simulink model "TosAeb"  (R2021a)
settings: Solver=FixedStepDiscrete  StartTime=0.0  StopTime=inf  FixedStep=0.01  RelTol=1e-3
undefined symbols (not defined in the file or in its folder): ACCModSts, AccSts1, AEB_ObjectMovingOrientation, AEB_ObjectMovingState, CN_BTCActive, CN_CurrentGearR, CN_CurrentGearR_DHT, CN_PTCActive, CN_VDCActive, CN_VSC_EspOff, CN_VSC_FCW_AEB_Error, CN_VSC_FCW_AEB_NoDispay, CN_VSC_FCW_AEB_PerfmDgdtn, CN_VSC_FCW_AEB_TempUnAvlbl, CN_VSC_VehStandstill, CNaccelAvoidMax, CNaccelAvoidMin, CNbrakePedalPress, CNcomfortStrLeftAccelMax, CNcomfortStrLeftAccelMin, CNcomfortStrRightAccelMax, CNcomfortStrRightAccelMin, CNDrvDoorOpen, CNDrvModeEco, CNDrvModeNorm, CNDrvModeOther, CNDrvModeSport, CNDrvSBRreminder, CNNull, CNobjInvalidID, CNPassDoorOpen, CNPassSBRreminder, CNPEBSAccelNegInf, CNPEBSAccelNull, CNPEBSTimeInf, CNPEBSTimeNull, CNSTOPMAX, CNSTOPNULL, CNsubjWidth, CNSwtOFF, CNSwtON, CNTTCMAX, CNTTCNULL, CNVehConfigAEBCycNone, CNVehConfigAEBNone, CNVehConfigAEBPedNone, CNVehConfigFCWNone, CNVehConfigJANone, CollisionSeverity, FALSE, FCWSnvty, JACycObjCalcInfoDefaultParams, JAPedObjCalcInfoDefaultParams, JAVehObjCalcInfoDefaultParams, latCycObjCalcInfoDefaultParams, latPedObjCalcInfoDefaultParams, longObjCalcInfoDefaultParams, ModSts, movingStatus, ObjectType ... (+1112)
libraries: simulink x3031, AEBSFunctionLib x50, Ta_Library x33, AEBSStateMachineLib x23, Mylibrary x12, simulink_extras x7, ObjSelect_lib x3, dspstat3 x2
(part 49 of 102)

== system TosAeb/FcwAebJa_Step_sys/ALG_AEBSystem/ALG_AEBController/AEB_LongTgtInfoCalc/LongObjCalcInfoCalc/AEBS_LongCollisionAssesApp/DriverReactionTimeCalc/DriverStateMonitor ==
  BrakePedalStatus_G : Inport
  ESP_MasterCylindBrakePress : Inport  Port=2
  AccrPedlPosnDiagc : Inport  Port=3
  SteerWheelSpd : Inport  Port=4
  LeftTurnSWSts : Inport  Port=5
  RightTurnSWSts : Inport  Port=6
  Abs : Abs
  Compare To Constant4 : simulink/Logic and Bit Operations/Compare To Constant  relop===  const=1  OutDataTypeStr=boolean
  Compare To Constant5 : simulink/Logic and Bit Operations/Compare To Constant  relop===  const=0  OutDataTypeStr=boolean
  Compare To Constant6 : simulink/Logic and Bit Operations/Compare To Constant  relop===  const=0  OutDataTypeStr=boolean
  Discrete Derivative : simulink/Discrete/Discrete Derivative  gainval=1.0  ICPrevScaledInput=0.0  OutMin=[]  OutMax=[]  OutDataType=fixdt(1, 16)  OutScaling=2^-10  DoSatur=off
  Discrete Derivative1 : simulink/Discrete/Discrete Derivative  gainval=1.0  ICPrevScaledInput=0.0  OutMin=[]  OutMax=[]  OutDataType=fixdt(1, 16)  OutScaling=2^-10  DoSatur=off
  Discrete Derivative2 : simulink/Discrete/Discrete Derivative  gainval=1.0  ICPrevScaledInput=0.0  OutMin=[]  OutMax=[]  OutDataType=fixdt(1, 16)  OutScaling=2^-10  DoSatur=off
  Logical Operator : Logic  AllPortsSameDT=off  OutDataTypeStr=boolean
  Logical Operator1 : Logic  Operator=OR  AllPortsSameDT=off  OutDataTypeStr=boolean
  Logical Operator2 : Logic  Operator=OR  Inputs=4  AllPortsSameDT=off  OutDataTypeStr=boolean
  Logical Operator3 : Logic  Operator=OR  AllPortsSameDT=off  OutDataTypeStr=boolean
  Logical Operator4 : Logic  AllPortsSameDT=off  OutDataTypeStr=boolean
  Logical Operator5 : Logic  AllPortsSameDT=off  OutDataTypeStr=boolean
  P_LongCA_MasterCylinderPresSlopThres : simulink/Logic and Bit Operations/Compare To Constant  relop=>  const=P_AEB_LongCA_MasterCylinderPresSlopThres  OutDataTypeStr=boolean
  P_LongCA_ReleaseGasPedalSlopThes : simulink/Logic and Bit Operations/Compare To Constant  relop=<  const=P_AEB_LongCA_ReleaseGasPedalSlopThes  OutDataTypeStr=boolean
  P_LongCA_StepGasPedalSlopThes : simulink/Logic and Bit Operations/Compare To Constant  relop=>  const=P_AEB_LongCA_StepGasPedalSlopThes  OutDataTypeStr=boolean
  P_LongCA_StrWheelSpdThes : simulink/Logic and Bit Operations/Compare To Constant  relop=>  const=P_AEB_LongCA_StrWheelSpdThes  OutDataTypeStr=boolean
  Unit Delay : UnitDelay  SampleTime=-1  HasFrameUpgradeWarning=on
  Unit Delay1 : UnitDelay  SampleTime=-1  HasFrameUpgradeWarning=on
  driverMonitor : Outport
  wires:
  ESP_MasterCylindBrakePress/1 -> Discrete Derivative/1
  P_LongCA_MasterCylinderPresSlopThres/1 -> Logical Operator/2
  Compare To Constant4/1 -> Logical Operator/1
  Discrete Derivative/1 -> P_LongCA_MasterCylinderPresSlopThres/1  "PMCPBChangeRate"
  BrakePedalStatus_G/1 -> Compare To Constant4/1
  P_LongCA_StepGasPedalSlopThes/1 -> Logical Operator1/1
  Discrete Derivative1/1 -> P_LongCA_StepGasPedalSlopThes/1, Abs/1  "thrtlPosChangeRate"
  P_LongCA_ReleaseGasPedalSlopThes/1 -> Logical Operator1/2
  AccrPedlPosnDiagc/1 -> Discrete Derivative1/1
  Logical Operator1/1 -> Logical Operator2/2  "DriverActiveThrtlFlag"
  Logical Operator/1 -> Logical Operator2/1  "DriverAttentiveByGasPedalFlag"
  Logical Operator2/1 -> driverMonitor/1
  Discrete Derivative2/1 -> P_LongCA_StrWheelSpdThes/1  "strWhelAnglChangeRate"
  SteerWheelSpd/1 -> Discrete Derivative2/1
  P_LongCA_StrWheelSpdThes/1 -> Logical Operator2/3  "DriverActiveSteeringFlag"
  Compare To Constant5/1 -> Logical Operator4/2
  Logical Operator5/1 -> Logical Operator3/2
  Unit Delay/1 -> Compare To Constant5/1
  Compare To Constant6/1 -> Logical Operator5/2
  Unit Delay1/1 -> Compare To Constant6/1
  Logical Operator4/1 -> Logical Operator3/1
  Logical Operator3/1 -> Logical Operator2/4  "DriverActiveDetectionIndicatorFlag"
  LeftTurnSWSts/1 -> Unit Delay/1, Logical Operator4/1
  RightTurnSWSts/1 -> Unit Delay1/1, Logical Operator5/1
  Abs/1 -> P_LongCA_ReleaseGasPedalSlopThes/1

== system TosAeb/FcwAebJa_Step_sys/ALG_AEBSystem/ALG_AEBController/AEB_LongTgtInfoCalc/LongObjCalcInfoCalc/AEBS_LongCollisionAssesApp/DriverReactionTimeCalc/ReationTimeCalc1 ==
  FcwHMISetting : Inport
  ACCMonitor : Inport  Port=2  OutDataTypeStr=boolean
  driverMonitor : Inport  Port=3  OutDataTypeStr=boolean
  Bus Creator : BusCreator  Inputs=2
  Constant : Constant  Value=P_AEB_LongCA_ReactionTime4WarnDrvrAtntvHmiEarly
  Constant1 : Constant  Value=P_AEB_LongCA_ReactionTime4WarnDrvrAtntvHmiMid
  Constant2 : Constant  Value=P_AEB_LongCA_ReactionTime4WarnDrvrAtntvHmiLate
  Constant3 : Constant  Value=P_AEB_LongCA_ReactionTime4WarnDrvrInatntvHmiEarly
  Constant4 : Constant  Value=P_AEB_LongCA_ReactionTime4WarnDrvrInatntvHmiMid
  Constant5 : Constant  Value=P_AEB_LongCA_ReactionTime4WarnDrvrInatntvHmiLate
  Constant6 : Constant  Value=1.5  OutDataTypeStr=single
  Constant7 : Constant  Value=0.9  OutDataTypeStr=single
  Constant8 : Constant  Value=P_AEB_LongCA_ReactionTime4BrkDrvrAtntv
  Constant9 : Constant  Value=P_AEB_LongCA_ReactionTime4BrkDrvrInatntv
  Logical Operator4 : Logic  Operator=OR  AllPortsSameDT=off  OutDataTypeStr=boolean
  ReactionTimeLevel1Calc : Stateflow chart  TreatAsAtomicUnit=on
  Switch4 : Switch  Criteria=u2 ~= 0
  Terminator : Terminator
  Terminator1 : Terminator
  driverReactionTime : Outport
  wires:
  Logical Operator4/1 -> ReactionTimeLevel1Calc/2(driverDetectAsActiveFlag), Switch4/2  "driverDetectAsActiveFlg"
  FcwHMISetting/1 -> ReactionTimeLevel1Calc/1(FcwHMISetting)
  driverMonitor/1 -> Logical Operator4/2
  ACCMonitor/1 -> Logical Operator4/1
  Constant8/1 -> Switch4/1
  Constant9/1 -> Switch4/3
  Constant/1 -> ReactionTimeLevel1Calc/3(PreactionTimeLvl1HMIEarly)
  Constant1/1 -> ReactionTimeLevel1Calc/4(PreactionTimeLvl1HMIMiddle)
  Constant2/1 -> ReactionTimeLevel1Calc/5(PreactionTimeLvl1HMILate)
  Constant3/1 -> ReactionTimeLevel1Calc/6(PreactionTimeAttentiveDriverLvl1HMIEarly)
  Constant4/1 -> ReactionTimeLevel1Calc/7(PreactionTimeAttentiveDriverLvl1HMIMiddle)
  Constant5/1 -> ReactionTimeLevel1Calc/8(PreactionTimeAttentiveDriverLvl1HMILate)
  Bus Creator/1 -> driverReactionTime/1  "driverReactionTime"
  Constant6/1 -> Bus Creator/1  "driverReactionTimeLvl1"
  Constant7/1 -> Bus Creator/2  "driverReactionTimeLvl2"
  ReactionTimeLevel1Calc/1(diverReactionTimeLvl1) -> Terminator/1  "driverReactionTimeLvl1"
  Switch4/1 -> Terminator1/1  "driverReactionTimeLvl2"

== system TosAeb/FcwAebJa_Step_sys/ALG_AEBSystem/ALG_AEBController/AEB_LongTgtInfoCalc/LongObjCalcInfoCalc/AEBS_LongCollisionAssesApp/LatCollisionAvoidCalc/AccelLatAvoidCalc4SO ==
  dy : Inport
  dxFrontBumper : Inport  Port=2
  subjVel : Inport  Port=3
  Action Port : ActionPort  ActionPortLabel=if(u1)
  Bus Creator : BusCreator  Inputs=2
  accelLatAvoidLeftCalc : Subsystem
  accelLatAvoidRightCalc : Subsystem
  accelLatAvoid : Outport
  wires:
  dxFrontBumper/1 -> accelLatAvoidLeftCalc/2(dxRelTargetObjCalc), accelLatAvoidRightCalc/2(dxRelTargetObjCalc)
  subjVel/1 -> accelLatAvoidLeftCalc/3(subjVel), accelLatAvoidRightCalc/3(subjVel)
  dy/1 -> accelLatAvoidLeftCalc/1(dyRelTargetObjCalc), accelLatAvoidRightCalc/1(dyRelTargetObjCalc)
  Bus Creator/1 -> accelLatAvoid/1
  accelLatAvoidLeftCalc/1(accelLatAvoidLeft) -> Bus Creator/1  "accelLatAvoidLeft"
  accelLatAvoidRightCalc/1(accelLatAvoidRight) -> Bus Creator/2  "accelLatAvoidRight"

== system TosAeb/FcwAebJa_Step_sys/ALG_AEBSystem/ALG_AEBController/AEB_LongTgtInfoCalc/LongObjCalcInfoCalc/AEBS_LongCollisionAssesApp/LatCollisionAvoidCalc/AccelLatAvoidCalcMO ==
  dxFrontBumper : Inport
  vx : Inport  Port=2
  TTC : Inport  Port=3
  dy : Inport  Port=4
  vy : Inport  Port=5
  Action Port : ActionPort  ActionPortLabel=else
  MovingObjLatCollisionAvoidCalc : Subsystem
  accelLatAvoid : Outport
  wires:
  MovingObjLatCollisionAvoidCalc/1(accelLatAvoid) -> accelLatAvoid/1
  vy/1 -> MovingObjLatCollisionAvoidCalc/5(vy)
  dy/1 -> MovingObjLatCollisionAvoidCalc/4(dy)
  TTC/1 -> MovingObjLatCollisionAvoidCalc/3(TTC)
  vx/1 -> MovingObjLatCollisionAvoidCalc/2(vx)
  dxFrontBumper/1 -> MovingObjLatCollisionAvoidCalc/1(dxFrontBumper)

== system TosAeb/FcwAebJa_Step_sys/ALG_AEBSystem/ALG_AEBController/AEB_LongTgtInfoCalc/LongObjCalcInfoCalc/AEBS_LongCollisionAssesApp/LatCollisionAvoidCalc/LatAvoidFlg4BrakeJudge ==
  note: if (comfortStrAccelLeft >  comfortStrThres) ||
      |    (comfortStrAccelRight< abs(comfortStrThres) )
      | 	comfortStrFlg4Brake = true;
      | else
      | 	comfortStrFlg4Brake = false;
  accelLatAvoidLeft : Inport
  accelLatAvoidRight : Inport  Port=2
  subjVel : Inport  Port=3
  Abs : Abs
  Logical Operator : Logic  Operator=OR  AllPortsSameDT=off  OutDataTypeStr=boolean
  Relational Operator : RelationalOperator  Operator=>  OutDataTypeStr=boolean  RndMeth=Simplest
  Relational Operator1 : RelationalOperator  Operator=<  OutDataTypeStr=boolean  RndMeth=Simplest
  latAvoidFlg4BrakeLookupTable : Lookup_n-D  NumberOfTableDimensions=1  BreakpointsForDimension1=P_AEB_LongCA_LatAvoidAccelThesBrkX  BeginIndexSearchUsingPreviousIndexResult=on  Table=P_AEB_LongCA_LatAvoidAccelThesBrkY  ExtrapMethod=Clip  InternalRulePriority=Speed  RndMeth=Simplest  InputSameDT=on
  latAvoidFlg4Brake : Outport
  wires:
  accelLatAvoidLeft/1 -> Relational Operator1/1
  Relational Operator1/1 -> Logical Operator/1
  Logical Operator/1 -> latAvoidFlg4Brake/1
  Relational Operator/1 -> Logical Operator/2
  latAvoidFlg4BrakeLookupTable/1 -> Relational Operator/2, Abs/1  "AEB_LongCA_LatAvoidAccelThesBrk"
  Abs/1 -> Relational Operator1/2
  accelLatAvoidRight/1 -> Relational Operator/1
  subjVel/1 -> latAvoidFlg4BrakeLookupTable/1

== system TosAeb/FcwAebJa_Step_sys/ALG_AEBSystem/ALG_AEBController/AEB_LongTgtInfoCalc/LongObjCalcInfoCalc/AEBS_LongCollisionAssesApp/LatCollisionAvoidCalc/LatAvoidFlg4WarningJudge ==
  ObjectMovingState : Inport
  FcwHMISetting : Inport  Port=2
  subjVel : Inport  Port=3
  accelLatAvoidLeft : Inport  Port=4
  accelLatAvoidRight : Inport  Port=5
  If : If  IfExpression=u1
  Merge1 : Merge
  ObjIsStillJudge : Subsystem
  accelLatAvoidThesMO : Subsystem  TreatAsAtomicUnit=on
  accelLatAvoidThesSO : Subsystem  TreatAsAtomicUnit=on
  latAvoidFlg4WarningCalc : Subsystem
  latAvoidFlg4Warning : Outport
  wires:
  If/1 -> accelLatAvoidThesSO/Action
  ObjectMovingState/1 -> ObjIsStillJudge/1(ObjectMovingState)
  ObjIsStillJudge/1(StillFlg) -> If/1
  accelLatAvoidThesSO/1(accelLatAvoidThes) -> Merge1/1
  accelLatAvoidThesMO/1(accelLatAvoidThes) -> Merge1/2
  subjVel/1 -> accelLatAvoidThesMO/2(subjVel), accelLatAvoidThesSO/2(subjVel)
  FcwHMISetting/1 -> accelLatAvoidThesSO/1(FcwHMISetting), accelLatAvoidThesMO/1(FcwHMISetting)
  If/2 -> [ObjMoving]
  [ObjMoving] -> accelLatAvoidThesMO/Action
  accelLatAvoidLeft/1 -> latAvoidFlg4WarningCalc/1(accelLatAvoidLeft)
  accelLatAvoidRight/1 -> latAvoidFlg4WarningCalc/2(accelLatAvoidRight)
  Merge1/1 -> latAvoidFlg4WarningCalc/3(accelLatAvoidThes)  "AEB_LongCA_LatAvoidAccelThesWarn"
  latAvoidFlg4WarningCalc/1(latAvoidFlg4Warning) -> latAvoidFlg4Warning/1

== system TosAeb/FcwAebJa_Step_sys/ALG_AEBSystem/ALG_AEBController/AEB_LongTgtInfoCalc/LongObjCalcInfoCalc/AEBS_LongCollisionAssesApp/LatCollisionAvoidCalc/ObjIsStationaryJudge ==
  ObjectMovingState : Inport
  Compare To Constant : simulink/Logic and Bit Operations/Compare To Constant  relop===  const=AEB_ObjectMovingState.Still  OutDataTypeStr=boolean
  objIsStationaryFlg : Outport
  wires:
  Compare To Constant/1 -> objIsStationaryFlg/1
  ObjectMovingState/1 -> Compare To Constant/1

== system TosAeb/FcwAebJa_Step_sys/ALG_AEBSystem/ALG_AEBController/AEB_LongTgtInfoCalc/LongObjCalcInfoCalc/AEBS_LongCollisionAssesApp/LongCollisionAvoidAssess/HighLvlBrkCalc ==
  tEgoStop : Inport
  tObjStop : Inport  Port=2
  TTC : Inport  Port=3
  tReaction : Inport  Port=4
  vx : Inport  Port=5
  axAbsObj : Inport  Port=6
  vxAbsObj : Inport  Port=7
  ax : Inport  Port=8
  subjLongAccel : Inport  Port=9
  dxFrontBumper : Inport  Port=10
  subjVel : Inport  Port=11
  Bus Creator1 : BusCreator  Inputs=11
  Bus Selector : BusSelector  OutputSignals=tEgoStop,tObjStop,TTC,tReaction.driverReactionTimeLvl1,vx,axAbsObj,vxAbsObj,ax,dxFrontBumper,subjVel,subjLongAccel
  Bus Selector1 : BusSelector  OutputSignals=tEgoStop,tObjStop,TTC,tReaction.driverReactionTimeLvl2,vx,axAbsObj,vxAbsObj,ax,dxFrontBumper,subjVel,subjLongAccel
  HighLvlBrkCalc4Brake : Subsystem  TreatAsAtomicUnit=on
  HighLvlBrkCalc4Warning : Subsystem  TreatAsAtomicUnit=on
  accelAvoidHighLvlBrk4Waning : Outport
  highLvlBrkFlg4Warning : Outport  Port=2
  accelAvoidHighLvlBrk4Brake : Outport  Port=3
  highLvlBrkFlg4Brake : Outport  Port=4
  wires:
  HighLvlBrkCalc4Brake/2(highLvlBrkFlg) -> highLvlBrkFlg4Brake/1  "highLvlBrkFlg4Brake"
  HighLvlBrkCalc4Brake/1(accelAvoidHighLvlBrk) -> accelAvoidHighLvlBrk4Brake/1  "accelAvoidHighLvlBrk4Brake"
  HighLvlBrkCalc4Warning/2(highLvlBrkFlg) -> highLvlBrkFlg4Warning/1  "highLvlBrkFlg4Warning"
  HighLvlBrkCalc4Warning/1(accelAvoidHighLvlBrk) -> accelAvoidHighLvlBrk4Waning/1  "accelAvoidHighLvlBrk4Warning"
  ax/1 -> Bus Creator1/8  "ax"
  Bus Selector/5 -> HighLvlBrkCalc4Warning/5(vx)  "<vx>"
  dxFrontBumper/1 -> Bus Creator1/10  "dxFrontBumper"
  Bus Selector1/7 -> HighLvlBrkCalc4Brake/7(vxAbsObj)  "<vxAbsObj>"
  Bus Selector1/5 -> HighLvlBrkCalc4Brake/5(vx)  "<vx>"
  Bus Selector1/6 -> HighLvlBrkCalc4Brake/6(axAbsObj)  "<axAbsObj>"
  Bus Selector/11 -> HighLvlBrkCalc4Warning/11(subjLongAccel)  "<subjLongAccel>"
  Bus Selector1/2 -> HighLvlBrkCalc4Brake/2(tObjStop)  "<tObjStop>"
  Bus Selector/3 -> HighLvlBrkCalc4Warning/3(TTC)  "<TTC>"
  Bus Selector1/11 -> HighLvlBrkCalc4Brake/11(subjLongAccel)  "<subjLongAccel>"
  Bus Selector/9 -> HighLvlBrkCalc4Warning/9(dxFrontBumper)  "<dxFrontBumper>"
  tEgoStop/1 -> Bus Creator1/1  "tEgoStop"
  Bus Selector1/3 -> HighLvlBrkCalc4Brake/3(TTC)  "<TTC>"
  Bus Selector1/1 -> HighLvlBrkCalc4Brake/1(tEgoStop)  "<tEgoStop>"
  Bus Selector1/9 -> HighLvlBrkCalc4Brake/9(dxFrontBumper)  "<dxFrontBumper>"
  Bus Selector1/8 -> HighLvlBrkCalc4Brake/8(ax)  "<ax>"
  tObjStop/1 -> Bus Creator1/2  "tObjStop"
  subjLongAccel/1 -> Bus Creator1/9  "subjLongAccel"
  Bus Selector/10 -> HighLvlBrkCalc4Warning/10(subjVel)  "<subjVel>"
  Bus Selector1/4 -> HighLvlBrkCalc4Brake/4(tReaction)  "<driverReactionTimeLvl2>"
  Bus Selector/8 -> HighLvlBrkCalc4Warning/8(ax)  "<ax>"
  Bus Selector/7 -> HighLvlBrkCalc4Warning/7(vxAbsObj)  "<vxAbsObj>"
  Bus Selector1/10 -> HighLvlBrkCalc4Brake/10(subjVel)  "<subjVel>"
  Bus Selector/1 -> HighLvlBrkCalc4Warning/1(tEgoStop)  "<tEgoStop>"
  Bus Selector/2 -> HighLvlBrkCalc4Warning/2(tObjStop)  "<tObjStop>"
  Bus Selector/4 -> HighLvlBrkCalc4Warning/4(tReaction)  "<driverReactionTimeLvl1>"
  Bus Selector/6 -> HighLvlBrkCalc4Warning/6(axAbsObj)  "<axAbsObj>"
  vxAbsObj/1 -> Bus Creator1/7  "vxAbsObj"
  TTC/1 -> Bus Creator1/3  "TTC"
  Bus Creator1/1 -> Bus Selector/1, Bus Selector1/1
  subjVel/1 -> Bus Creator1/11  "subjVel"
  tReaction/1 -> Bus Creator1/4  "tReaction"
  vx/1 -> Bus Creator1/5  "vx"
  axAbsObj/1 -> Bus Creator1/6  "axAbsObj"

== system TosAeb/FcwAebJa_Step_sys/ALG_AEBSystem/ALG_AEBController/AEB_LongTgtInfoCalc/LongObjCalcInfoCalc/AEBS_LongCollisionAssesApp/LongCollisionAvoidAssess/LowLvlBrkCalc ==
  note: 修改信息：
      | 1. dxEgoBrakeCalc模块增加限幅模块，防止减速度计算出现除零错误
      | 2. 修改 计算accelAvoid模块中场景调度模块，减少复杂度和死逻辑，对于oncoming被选入的情况，归结到不计算AccelAvoid，即AccelVoid为0.
      | 3. 修改AccelerateAvoid计算Situation1 模块中TTC限幅模块，取消最小值的限制（dxEgoBrake和Vx计算出的TTC无小于0的情况）
  vx : Inport
  axAbsObj : Inport  Port=2
  vxAbsObj : Inport  Port=3
  dxFrontBumper : Inport  Port=4
  subjVel : Inport  Port=5
  FcwHMISetting : Inport  Port=6
  tObjStop : Inport  Port=7
  LowLvlBrkFlg4BrakeJudge : Subsystem  TreatAsAtomicUnit=on
  LowLvlBrkFlg4WarningJudge : Subsystem  TreatAsAtomicUnit=on
  accelAvoidLowLvlBrkCalc : Subsystem  TreatAsAtomicUnit=on
  dxEgoBrakeCalc : Subsystem  TreatAsAtomicUnit=on
  accelAvoidLowLvlBrk : Outport
  lowLvlBrkFlg4Warning : Outport  Port=2
  lowLvlBrkFlg4Brake : Outport  Port=3
  wires:
  vxAbsObj/1 -> accelAvoidLowLvlBrkCalc/3(vxAbsObj)
  LowLvlBrkFlg4BrakeJudge/1(lowLvlBrkFlg4Brake) -> lowLvlBrkFlg4Brake/1  "lowLvlBrkFlg4Brake"
  LowLvlBrkFlg4WarningJudge/1(lowLvlBrkFlg4Warning) -> lowLvlBrkFlg4Warning/1  "comfortBrkFlg4Warning"
  vx/1 -> accelAvoidLowLvlBrkCalc/1(vx), dxEgoBrakeCalc/2(vx)
  tObjStop/1 -> accelAvoidLowLvlBrkCalc/5(tObjStop)
  [subjVel] -> LowLvlBrkFlg4BrakeJudge/2(subjVel)  "subjVel"
  dxFrontBumper/1 -> dxEgoBrakeCalc/1(dxFrontBumper)
  accelAvoidLowLvlBrkCalc/1(accelAvoidLowLvlBrk) -> accelAvoidLowLvlBrk/1, LowLvlBrkFlg4BrakeJudge/1(accelAvoidLowLvlBrk), LowLvlBrkFlg4WarningJudge/2(accelAvoidLowLvlBrk)  "accelAvoidLowLvlBrk"
  subjVel/1 -> accelAvoidLowLvlBrkCalc/4(subjVel), [subjVel]
  [subjVel] -> LowLvlBrkFlg4WarningJudge/3(subjVel)  "subjVel"
  axAbsObj/1 -> accelAvoidLowLvlBrkCalc/2(axAbsObj)
  FcwHMISetting/1 -> LowLvlBrkFlg4WarningJudge/1(FcwHMISetting)  "FcwHMISetting"
  dxEgoBrakeCalc/1(distEgoBrake) -> accelAvoidLowLvlBrkCalc/6(dxEgoBrake)

== system TosAeb/FcwAebJa_Step_sys/ALG_AEBSystem/ALG_AEBController/AEB_LongTgtInfoCalc/LongObjCalcInfoCalc/AEBS_LongCollisionAssesApp/TTCCalc/TTCCalc ==
  note: NOTE：修改信息
      | 修改situation2 和situation3 接口连接错误
      | 修改tEgoStop调用形式
  note: tObjStopCalc
  subjLongAccel : Inport
  subjVel : Inport  Port=2
  axAbsObj : Inport  Port=3
  vxAbsObj : Inport  Port=4
  ax : Inport  Port=5
  vx : Inport  Port=6
  dxFrontBumper : Inport  Port=7
  Action Port : ActionPort  ActionPortLabel=else
  Bus Creator : BusCreator  Inputs=7
  Bus Creator1 : BusCreator  Inputs=3
  Bus Selector : BusSelector  OutputSignals=subjLongAccel,subjVel
  Bus Selector1 : BusSelector  OutputSignals=axAbsObj,vxAbsObj
  Bus Selector2 : BusSelector  OutputSignals=ax,vx,dxFrontBumper
  Bus Selector3 : BusSelector  OutputSignals=subjLongAccel,subjVel,dxFrontBumper,axAbsObj,vxAbsObj
  Bus Selector4 : BusSelector  OutputSignals=subjLongAccel,subjVel,axAbsObj,dxFrontBumper,vxAbsObj
  Bus Selector5 : BusSelector  OutputSignals=vx,dxFrontBumper
  Situation2 : Subsystem
  Situation3 : Subsystem
  SloveTTCQuadraticEquation : Subsystem  TreatAsAtomicUnit=on
  TTCCalc : Stateflow chart  TreatAsAtomicUnit=on
  TTCFinalCalc : Subsystem  Commented=on
  TimegapCalc : Subsystem  TreatAsAtomicUnit=on
  tEgoStopCalc : Subsystem  TreatAsAtomicUnit=on
  tObjStopCalc : Subsystem  TreatAsAtomicUnit=on
  TTCCalcOut : Outport
  wires:
  SloveTTCQuadraticEquation/1(TTC) -> TTCCalc/3(rawTTC)
  tObjStopCalc/1(tStop) -> [tObjStop], TTCCalc/2(tObjStop)  "tObjStop"
  tEgoStopCalc/1(tStop) -> TTCCalc/1(tEgoStop), [tEgoStop]  "tEgoStop"
  [tEgoStop] -> Bus Creator1/2  "tEgoStop"
  [tObjStop] -> Bus Creator1/3  "tObjStop"
  TTCCalc/3(situation3()) -> [Situation3]
  [Situation3] -> Situation3/Trigger
  [tEgoStop] -> Situation2/6(tEgoStop)  "tEgoStop"
  [tObjStop] -> Situation2/7(tObjStop)  "tObjStop"
  [tEgoStop] -> Situation3/6(tEgoStop)
  [tObjStop] -> Situation3/7(tObjStop)
  subjLongAccel/1 -> Bus Creator/1  "subjLongAccel"
  subjVel/1 -> Bus Creator/2  "subjVel"
  axAbsObj/1 -> Bus Creator/3  "axAbsObj"
  vxAbsObj/1 -> Bus Creator/4  "vxAbsObj"
  ax/1 -> Bus Creator/5  "ax"
  vx/1 -> Bus Creator/6  "vx"
  dxFrontBumper/1 -> Bus Creator/7  "dxFrontBumper"
  Bus Selector/1 -> tEgoStopCalc/1(accelAbs)  "<subjLongAccel>"
  Bus Selector/2 -> tEgoStopCalc/2(velAbs)  "<subjVel>"
  Bus Creator/1 -> Bus Selector2/1, Bus Selector3/1, Bus Selector4/1, Bus Selector1/1, Bus Selector/1, Bus Selector5/1
  Bus Selector1/1 -> tObjStopCalc/1(accelAbs)  "<axAbsObj>"
  Bus Selector1/2 -> tObjStopCalc/2(velAbs)  "<vxAbsObj>"
  Bus Selector2/2 -> SloveTTCQuadraticEquation/2(velRel)  "<vx>"
  Bus Selector2/1 -> SloveTTCQuadraticEquation/1(accelRel)  "<ax>"
  Bus Selector2/3 -> SloveTTCQuadraticEquation/3(distRel)  "<dxFrontBumper>"
  Bus Selector3/1 -> Situation2/1(subjLongAccel)  "<subjLongAccel>"
  Bus Selector3/2 -> Situation2/2(subjVel)  "<subjVel>"
  Bus Selector3/3 -> Situation2/3(dxFrontBumper)  "<dxFrontBumper>"
  Bus Selector3/4 -> Situation2/4(axAbsObj)  "<axAbsObj>"
  Bus Selector3/5 -> Situation2/5(vxAbsObj)  "<vxAbsObj>"
  Bus Selector4/4 -> Situation3/4(dxFrontBumper)  "<dxFrontBumper>"
  Bus Selector4/3 -> Situation3/3(axAbsObj)  "<axAbsObj>"
  Bus Selector4/5 -> Situation3/5(vxAbsObj)  "<vxAbsObj>"
  Bus Selector4/1 -> Situation3/1(subjLongAccel)  "<subjLongAccel>"
  Bus Selector4/2 -> Situation3/2(subjVel)  "<subjVel>"
  Bus Creator1/1 -> TTCCalcOut/1
  TTCCalc/2(situation2()) -> [Situation2]
  [Situation2] -> Situation2/Trigger
  Situation2/1(TTCOut1) -> TTCCalc/4(TTCIn1)
  Situation3/1(TTCOut2) -> TTCCalc/5(TTCIn2)
  Bus Selector5/1 -> TimegapCalc/1(vx)  "<vx>"
  Bus Selector5/2 -> TimegapCalc/2(dxFrontBumper)  "<dxFrontBumper>"
  TimegapCalc/1(Timegap) -> TTCFinalCalc/1(Timegap)  "Timegap"
  TTCCalc/1(TTC) -> Bus Creator1/1  "TTC"

== system TosAeb/FcwAebJa_Step_sys/ALG_AEBSystem/ALG_AEBController/AEB_LongTgtInfoCalc/LongObjCalcInfoCalc/AEBS_LongCollisionAssesApp/TTCCalc/TTCNull ==
  Action Port : ActionPort  ActionPortLabel=if(u1 <= 0)
  Bus Creator : BusCreator  Inputs=3
  Constant : Constant  Value=CNNull
  Constant1 : Constant  Value=CNNull
  Constant2 : Constant  Value=CNNull
  TTCCalcOut : Outport
  wires:
  Constant/1 -> Bus Creator/1  "TTC"
  Constant1/1 -> Bus Creator/2  "tEgoStop"
  Constant2/1 -> Bus Creator/3  "tObjStop"
  Bus Creator/1 -> TTCCalcOut/1

== system TosAeb/FcwAebJa_Step_sys/ALG_AEBSystem/ALG_AEBController/AEB_LongTgtInfoCalc/LongObjCalcInfoCalc/AEBS_LongCollisionAssesApp/collisionSeverityHighCalc/collisionSeverityHighCalc1 ==
  ObjectMovingState : Inport
  subjLongAccel : Inport  Port=2
  vxAbsObj : Inport  Port=3
  axAbsObj : Inport  Port=4
  subjVel : Inport  Port=5
  dxFrontBumper : Inport  Port=6
  vx : Inport  Port=7
  FCWSnvty : Inport  Port=8
  collisionDgrLvl2False : ActionPort  ActionPortLabel=else
  BrkSysAtribution : Subsystem
  Bus Selector : BusSelector  OutputSignals=accelGradient,accelMaxReudce,velMaxReudce
  CollisionAssessHighCalcVersion3 : Subsystem
  dxSecureCalc : Subsystem
  collisionSeverityHighFlg : Outport
  wires:
  vx/1 -> dxSecureCalc/1(vx)  "vx"
  ObjectMovingState/1 -> BrkSysAtribution/2(ObjectMovingState)  "ObjectMovingState"
  CollisionAssessHighCalcVersion3/1(collisionSeverityHighFlg) -> collisionSeverityHighFlg/1  "collisionSeverityHighFlg"
  dxFrontBumper/1 -> CollisionAssessHighCalcVersion3/9(dxFrontBumper)  "dxFrontBumper"
  dxSecureCalc/1(dxSecure) -> CollisionAssessHighCalcVersion3/10(dxSecure)  "dxSecure"
  subjLongAccel/1 -> CollisionAssessHighCalcVersion3/5(subjLongAccel), BrkSysAtribution/4(subjLongAccel)  "subjLongAccel"
  subjVel/1 -> BrkSysAtribution/3(subjVel), CollisionAssessHighCalcVersion3/8(subjVel)  "subjVel"
  axAbsObj/1 -> CollisionAssessHighCalcVersion3/7(axAbsObj)  "axAbsObj"
  vxAbsObj/1 -> CollisionAssessHighCalcVersion3/6(vxAbsObj)  "vxAbsObj"
  BrkSysAtribution/1(brkSysAtribution) -> Bus Selector/1  "brkSysAtribution"
  Bus Selector/2 -> CollisionAssessHighCalcVersion3/2(accelMaxDec)  "<accelMaxReudce>"
  Bus Selector/1 -> CollisionAssessHighCalcVersion3/1(accelGradient)  "<accelGradient>"
  Bus Selector/3 -> CollisionAssessHighCalcVersion3/3(velMaxReudce)  "<velMaxReudce>"
  BrkSysAtribution/2(tBrakeLatency) -> CollisionAssessHighCalcVersion3/4(tBrakeLatency)  "AEB_LongCA_SeverityHighDlyTime"
  FCWSnvty/1 -> BrkSysAtribution/1(FCWSnvty)

== system TosAeb/FcwAebJa_Step_sys/ALG_AEBSystem/ALG_AEBController/AEB_LongTgtInfoCalc/LongObjCalcInfoCalc/AEBS_LongCollisionAssesApp/collisionSeverityHighCalc/collisionSeverityHighCalc2 ==
  ObjectMovingState : Inport
  vx : Inport  Port=2
  dxFrontBumper : Inport  Port=3
  ax : Inport  Port=4
  vxAbsObj : Inport  Port=5
  axAbsObj : Inport  Port=6
  subjLongAccel : Inport  Port=7
  subjVel : Inport  Port=8
  collisionDgrLvl2False : ActionPort
  BrkSysAtribution : Subsystem
  Bus Creator : BusCreator  Inputs=9
  Bus Selector : BusSelector  OutputSignals=tWorkPhase1,distTargetPhase1,distEgoPhase1
  Bus Selector1 : BusSelector  OutputSignals=collisionSeverityHighFlgPhase1
  Bus Selector2 : BusSelector  OutputSignals=collisionSeverityHighFlgPhase2
  Bus Selector3 : BusSelector  OutputSignals=EgoObjDisVelAccelAfterPhase1.subjVelWorkPhase1,tWorkPhase2,EgoObjDisVelAccelAfterPhase1.axAbsWorkPhase1Target,EgoObjDisVelAccelAfterPhase1.subjAccelWorkPhase1,EgoObjDisVelAccelAfterPhase1.vxAbsWorkPhase1Target,distTargetphase2,EgoObjDisVelAccelAfterPhase1.distRelWorkPhase1,distEgophase2
  Bus Selector4 : BusSelector  OutputSignals=brkSysAtribution.velMaxReudce,dxFrontBumper,subjLongAccel,subjVel,ax,vxAbsObj,axAbsObj,vx,dxSecure
  Bus Selector5 : BusSelector  OutputSignals=subjVel,subjLongAccel,vxAbsObj,axAbsObj,dxFrontBumper,brkSysAtribution.velMaxReudce,brkSysAtribution.accelGradient,dxSecure,brkSysAtribution.accelMaxReudce
  Bus Selector6 : BusSelector  OutputSignals=brkSysAtribution.accelGradient,brkSysAtribution.accelMaxReudce,dxSecure,brkSysAtribution.velMaxReudce
  collisionSeverityHighFlgJudge : Subsystem
  collisionSeverityHighPhase1 : Subsystem  TreatAsAtomicUnit=on
  collisionSeverityHighPhase2 : Subsystem  TreatAsAtomicUnit=on
  collisionSeverityHighPhase3 : Subsystem  TreatAsAtomicUnit=on
  dxSecureCalc : Subsystem
  collisionSeverityHighFlg : Outport
  wires:
  collisionSeverityHighPhase3/1(collisionSeverityHighPhase3) -> collisionSeverityHighFlgJudge/3(collisionSeverityHighFlgPhase3)
  collisionSeverityHighPhase1/1(collisionSeverityHighPhase1Out) -> Bus Selector1/1, Bus Selector/1
  collisionSeverityHighFlgJudge/1(collisionSeverityHighFlg) -> collisionSeverityHighFlg/1  "collisionDgrLvl3Fullfilled"
  Bus Selector/1 -> collisionSeverityHighPhase2/1(tWorkphase1)  "<tWorkPhase1>"
  Bus Selector/2 -> collisionSeverityHighPhase2/2(distTargetphase1)  "<distTargetPhase1>"
  Bus Selector/3 -> collisionSeverityHighPhase2/3(distEgophase1)  "<distEgoPhase1>"
  Bus Selector1/1 -> collisionSeverityHighFlgJudge/1(collisionSeverityHighFlgPhase1)  "<collisionSeverityHighFlgPhase1>"
  Bus Selector2/1 -> collisionSeverityHighFlgJudge/2(collisionSeverityHighFlgPhase2)  "<collisionSeverityHighFlgPhase2>"
  collisionSeverityHighPhase2/1(collisionSeverityHighPhase2Out) -> Bus Selector3/1, Bus Selector2/1
  Bus Selector3/1 -> collisionSeverityHighPhase3/1(subjVelWorkPhase1)  "<subjVelWorkPhase1>"
  Bus Selector3/2 -> collisionSeverityHighPhase3/2("tWorkPhase2 ")  "<tWorkPhase2>"
  Bus Selector3/3 -> collisionSeverityHighPhase3/3(axAbsWorkPhase1Target)  "<axAbsWorkPhase1Target>"
  Bus Selector3/6 -> collisionSeverityHighPhase3/6(distTargetPhase2)  "<distTargetphase2>"
  Bus Selector3/7 -> collisionSeverityHighPhase3/7(dxRelWorkPhase1)  "<distRelWorkPhase1>"
  Bus Selector3/8 -> collisionSeverityHighPhase3/8(distEgoPhase2)  "<distEgophase2>"
  Bus Selector3/4 -> collisionSeverityHighPhase3/4(subjLongAccelWorkPhase1)  "<subjAccelWorkPhase1>"
  Bus Selector3/5 -> collisionSeverityHighPhase3/5(vxAbsWorkPhase1Target)  "<vxAbsWorkPhase1Target>"
  ObjectMovingState/1 -> BrkSysAtribution/1(ObjectMovingState)
  BrkSysAtribution/1(brkSysAtribution) -> Bus Creator/1  "brkSysAtribution"
  Bus Creator/1 -> Bus Selector6/1, Bus Selector5/1, Bus Selector4/1
  Bus Selector4/4 -> collisionSeverityHighPhase1/4(subjVel)  "<subjVel>"
  Bus Selector4/5 -> collisionSeverityHighPhase1/5(ax)  "<ax>"
  Bus Selector4/3 -> collisionSeverityHighPhase1/3(subjLongAccel)  "<subjLongAccel>"
  Bus Selector4/6 -> collisionSeverityHighPhase1/6(vxAbsObj)  "<vxAbsObj>"
  Bus Selector4/2 -> collisionSeverityHighPhase1/2(dxFrontBumper)  "<dxFrontBumper>"
  Bus Selector4/7 -> collisionSeverityHighPhase1/7(axAbsObj)  "<axAbsObj>"
  Bus Selector4/1 -> collisionSeverityHighPhase1/1(velMaxReudce)  "<velMaxReudce>"
  Bus Selector4/8 -> collisionSeverityHighPhase1/8(vx)  "<vx>"
  Bus Selector5/1 -> collisionSeverityHighPhase2/4(subjVel)  "<subjVel>"
  Bus Selector5/2 -> collisionSeverityHighPhase2/5(subjLongAccel)  "<subjLongAccel>"
  Bus Selector5/3 -> collisionSeverityHighPhase2/6(vxAbsObj)  "<vxAbsObj>"
  Bus Selector5/4 -> collisionSeverityHighPhase2/7(axAbsObj)  "<axAbsObj>"
  Bus Selector5/5 -> collisionSeverityHighPhase2/8(dxFrontBumper)  "<dxFrontBumper>"
  Bus Selector5/6 -> collisionSeverityHighPhase2/9(velMaxReduce)  "<velMaxReudce>"
  Bus Selector5/7 -> collisionSeverityHighPhase2/10(accelGradient)  "<accelGradient>"
  vx/1 -> dxSecureCalc/1(vx), Bus Creator/3
  dxSecureCalc/1(dxSecure) -> Bus Creator/2  "dxSecure"
  dxFrontBumper/1 -> Bus Creator/4  "dxFrontBumper"
  ax/1 -> Bus Creator/5  "ax"
  vxAbsObj/1 -> Bus Creator/6  "vxAbsObj"
  axAbsObj/1 -> Bus Creator/7  "axAbsObj"
  subjVel/1 -> Bus Creator/9  "subjVel"
  subjLongAccel/1 -> Bus Creator/8  "subjLongAccel"
  Bus Selector4/9 -> collisionSeverityHighPhase1/9(dxSecure)  "<dxSecure>"
  Bus Selector6/3 -> collisionSeverityHighPhase3/11(dxSecure)  "<dxSecure>"
  Bus Selector6/1 -> collisionSeverityHighPhase3/9(accelGradient)  "<accelGradient>"
  Bus Selector5/8 -> collisionSeverityHighPhase2/11(dxSecure)  "<dxSecure>"
  Bus Selector6/4 -> collisionSeverityHighPhase3/12(velMaxReudce)  "<velMaxReudce>"
  Bus Selector6/2 -> collisionSeverityHighPhase3/10(accelMaxReduce)  "<accelMaxReudce>"
  Bus Selector5/9 -> collisionSeverityHighPhase2/12(accelMaxReudce)  "<accelMaxReudce>"

== system TosAeb/FcwAebJa_Step_sys/ALG_AEBSystem/ALG_AEBController/AEB_LongTgtInfoCalc/LongObjCalcInfoCalc/AEBS_LongCollisionAssesApp/collisionSeverityHighCalc/collisionSeverityHighNoFullfilled ==
  collisionDgrLvl2False : ActionPort  ActionPortLabel=if(~u1)
  Constant : Constant  Value=FALSE  OutDataTypeStr=boolean
  collisionSeverityHighFlg : Outport
  wires:
  Constant/1 -> collisionSeverityHighFlg/1  "collisionSeverityHighFlg"

== system TosAeb/FcwAebJa_Step_sys/ALG_AEBSystem/ALG_AEBController/AEB_LongTgtInfoCalc/LongObjCalcInfoCalc/ProbEscapeCalcu/MovingObjdyEscapeCalcu/RadicalRelevantCalc ==
  dy : Inport
  dxFrontBumper : Inport  Port=2
  subjYawRate : Inport  Port=3
  Math Function : Math  Operator=hypot  SignedPower=on  SaturateOnIntegerOverflow=on
  Product1 : Product
  Trigonometric Function : Trigonometry  Operator=atan2
  radialVelRel : Outport
  objAngleToRear : Outport  Port=2
  wires:
  subjYawRate/1 -> Product1/2
  Math Function/1 -> Product1/1  "dvRadial"
  Product1/1 -> radialVelRel/1  "radialVelRel"
  Trigonometric Function/1 -> objAngleToRear/1
  dy/1 -> Trigonometric Function/1, Math Function/2
  dxFrontBumper/1 -> Trigonometric Function/2, Math Function/1

== system TosAeb/FcwAebJa_Step_sys/ALG_AEBSystem/ALG_AEBController/AEB_LongTgtInfoCalc/LongObjCalcInfoCalc/ProbEscapeCalcu/MovingObjdyEscapeCalcu/RadicalToXYTrans ==
  subjVel : Inport
  velRadicalXY : Inport  Port=2
  sincosCoef : Inport  Port=3
  Add3 : Sum  Inputs=++-
  Add4 : Sum
  Bus Creator : BusCreator  Inputs=2
  Bus Creator4 : BusCreator  Inputs=2
  Bus Selector10 : BusSelector  OutputSignals=velRadicalXY.velRadicalY
  Bus Selector11 : BusSelector  OutputSignals=sincosCoef.sinCoef
  Bus Selector12 : BusSelector  OutputSignals=velRadicalXY.velRadicalX
  Bus Selector13 : BusSelector  OutputSignals=sincosCoef.sinCoef
  Bus Selector14 : BusSelector  OutputSignals=velRadicalXY.velRadicalY
  Bus Selector15 : BusSelector  OutputSignals=sincosCoef.cosCoef
  Bus Selector8 : BusSelector  OutputSignals=velRadicalXY.velRadicalX
  Bus Selector9 : BusSelector  OutputSignals=sincosCoef.cosCoef
  Product{7..10} (4 alike) : Product
  velAbsTargetCorr : Outport
  wires:
  Product8/1 -> Add3/3
  Bus Selector13/1 -> Product9/2  "<sinCoef>"
  Add3/1 -> Bus Creator/1  "vxAbsTargetCorr"
  Product7/1 -> Add3/2
  Bus Selector14/1 -> Product10/1  "<velRadicalY>"
  Bus Selector11/1 -> Product8/2  "<sinCoef>"
  Bus Selector10/1 -> Product8/1  "<velRadicalY>"
  subjVel/1 -> Add3/1
  Add4/1 -> Bus Creator/2  "vyAbsTargetCorr"
  Bus Creator4/1 -> Bus Selector15/1, Bus Selector14/1, Bus Selector13/1, Bus Selector12/1, Bus Selector11/1, Bus Selector10/1, Bus Selector9/1, Bus Selector8/1
  Bus Selector9/1 -> Product7/2  "<cosCoef>"
  Product10/1 -> Add4/2
  Bus Selector15/1 -> Product10/2  "<cosCoef>"
  Bus Selector8/1 -> Product7/1  "<velRadicalX>"
  Product9/1 -> Add4/1
  Bus Selector12/1 -> Product9/1  "<velRadicalX>"
  velRadicalXY/1 -> Bus Creator4/1
  sincosCoef/1 -> Bus Creator4/2
  Bus Creator/1 -> velAbsTargetCorr/1

== system TosAeb/FcwAebJa_Step_sys/ALG_AEBSystem/ALG_AEBController/AEB_LongTgtInfoCalc/LongObjCalcInfoCalc/ProbEscapeCalcu/MovingObjdyEscapeCalcu/XYToRadicalTrans ==
  LongLatVelRel : Inport
  radialVelRel : Inport  Port=2
  sincosCoef : Inport  Port=3
  Add : Sum
  Add2 : Sum  Inputs=+-+
  Bus Creator2 : BusCreator  Inputs=2
  Bus Creator3 : BusCreator  Inputs=2
  Bus Selector : BusSelector  OutputSignals=LongLatVelRel.vx
  Bus Selector1 : BusSelector  OutputSignals=sincosCoef.cosCoef
  Bus Selector2 : BusSelector  OutputSignals=LongLatVelRel.vy
  Bus Selector3 : BusSelector  OutputSignals=sincosCoef.sinCoef
  Bus Selector4 : BusSelector  OutputSignals=LongLatVelRel.vy
  Bus Selector5 : BusSelector  OutputSignals=sincosCoef.cosCoef
  Bus Selector6 : BusSelector  OutputSignals=LongLatVelRel.vx
  Bus Selector7 : BusSelector  OutputSignals=sincosCoef.sinCoef
  Product{2,4..6} (4 alike) : Product
  velRadicalXY : Outport
  wires:
  Product6/1 -> Add2/2
  Bus Creator3/1 -> velRadicalXY/1  "velRadicalXY"
  Product5/1 -> Add2/1
  Bus Selector2/1 -> Product4/1  "<vy>"
  Product4/1 -> Add/2
  Product2/1 -> Add/1
  Bus Creator2/1 -> Bus Selector7/1, Bus Selector6/1, Bus Selector5/1, Bus Selector4/1, Bus Selector3/1, Bus Selector2/1, Bus Selector1/1, Bus Selector/1
  Bus Selector5/1 -> Product5/2  "<cosCoef>"
  Add2/1 -> Bus Creator3/2  "velRadicalY"
  Bus Selector6/1 -> Product6/1  "<vx>"
  Add/1 -> Bus Creator3/1  "velRadicalX"
  Bus Selector4/1 -> Product5/1  "<vy>"
  Bus Selector1/1 -> Product2/2  "<cosCoef>"
  radialVelRel/1 -> Add2/3
  Bus Selector3/1 -> Product4/2  "<sinCoef>"
  Bus Selector/1 -> Product2/1  "<vx>"
  Bus Selector7/1 -> Product6/2  "<sinCoef>"
  sincosCoef/1 -> Bus Creator2/2
  LongLatVelRel/1 -> Bus Creator2/1

== system TosAeb/FcwAebJa_Step_sys/ALG_AEBSystem/ALG_AEBController/AEB_LongTgtInfoCalc/LongObjCalcInfoCalc/ProbEscapeCalcu/MovingObjdyEscapeCalcu/dyEscapeFinalCalc ==
  dy : Inport
  dxFrontBumper : Inport  Port=2
  "subjCurvTraj " : Inport  Port=3
  vyAbsTargetFinal : Inport  Port=4
  TTC : Inport  Port=5
  Add6 : Sum
  Add7 : Sum  Inputs=+-+
  Constant : Constant  Value=0.5
  Math Function : Math  Operator=square  SignedPower=on  SaturateOnIntegerOverflow=on
  Math Function1 : Math  Operator=square  SignedPower=on  SaturateOnIntegerOverflow=on
  Product13 : Product
  Product16 : Product  Inputs=3
  dyEscape : Outport
  wires:
  Math Function1/1 -> Add6/2
  Product13/1 -> Add7/3
  Add6/1 -> Product16/1
  "subjCurvTraj "/1 -> Product16/2
  Constant/1 -> Product16/3
  Product16/1 -> Add7/2
  Add7/1 -> dyEscape/1  "dyEscape"
  vyAbsTargetFinal/1 -> Product13/1
  Math Function/1 -> Add6/1
  TTC/1 -> Product13/2
  dy/1 -> Add7/1, Math Function/1
  dxFrontBumper/1 -> Math Function1/1

== system TosAeb/FcwAebJa_Step_sys/ALG_AEBSystem/ALG_AEBController/AEB_LongTgtInfoCalc/LongObjCalcInfoCalc/ProbEscapeCalcu/MovingObjdyEscapeCalcu/sincosCoefCalc ==
  angle : Inport
  Bus Creator : BusCreator  Inputs=2
  Sincos : Trigonometry  Operator=sincos
  sincosCoef : Outport
  wires:
  Sincos/1 -> Bus Creator/1  "sinCoef"
  angle/1 -> Sincos/1
  Sincos/2 -> Bus Creator/2  "cosCoef"
  Bus Creator/1 -> sincosCoef/1  "sincosCoef"

== system TosAeb/FcwAebJa_Step_sys/ALG_AEBSystem/ALG_AEBController/AEB_LongTgtInfoCalc/LongObjCalcInfoCalc/ProbEscapeCalcu/MovingObjdyEscapeCalcu/vyAbsTargetFinalCalc ==
  velAbsTargetCorr : Inport
  subjYawRate : Inport  Port=2
  TTC : Inport  Port=3
  Add5 : Sum  Inputs=+-
  Bus Selector : BusSelector  OutputSignals=vyAbsTargetCorr,vxAbsTargetCorr
  Cos : Trigonometry  Operator=cos
  Product : Product
  Product11 : Product
  Product12 : Product
  Sin : Trigonometry
  vyAbsTargetFinal : Outport
  wires:
  TTC/1 -> Product/2
  subjYawRate/1 -> Product/1
  Product/1 -> Cos/1, Sin/1  "angleTTC"
  Cos/1 -> Product11/2
  Sin/1 -> Product12/1
  Add5/1 -> vyAbsTargetFinal/1  "vyAbsTargetFinal"
  Product11/1 -> Add5/1
  Product12/1 -> Add5/2
  velAbsTargetCorr/1 -> Bus Selector/1
  Bus Selector/1 -> Product11/1  "<vyAbsTargetCorr>"
  Bus Selector/2 -> Product12/2  "<vxAbsTargetCorr>"

== system TosAeb/FcwAebJa_Step_sys/ALG_AEBSystem/ALG_AEBController/AEB_LongTgtInfoCalc/LongObjCalcInfoCalc/ProbEscapeCalcu/Subsystem/TimegapCalc ==
  dxFrontBumper : Inport
  vx : Inport  Port=2
  Action Port : ActionPort  ActionPortLabel=else
  Abs : Abs
  Abs1 : Abs
  Divide : Product  Inputs=*/
  TTC : Outport
  wires:
  vx/1 -> Abs1/1
  dxFrontBumper/1 -> Abs/1
  Divide/1 -> TTC/1
  Abs/1 -> Divide/1
  Abs1/1 -> Divide/2

== system TosAeb/FcwAebJa_Step_sys/ALG_AEBSystem/ALG_AEBController/AEB_LongTgtInfoCalc/LongObjCalcInfoCalc/ProbEscapeCalcu/Subsystem/TimegapCalcDefault ==
  Action Port : ActionPort  ActionPortLabel=if(u1)
  Constant : Constant  Value=CNTTCMAX
  TTC : Outport
  wires:
  Constant/1 -> TTC/1

== system TosAeb/FcwAebJa_Step_sys/ALG_AEBSystem/ALG_AEBController/AEB_LongTgtInfoCalc/LongObjCalcInfoCalc/ProbEscapeCalcu/Subsystem/subjVelZeroFlgCalc ==
  subjVel : Inport
  Abs : Abs
  Compare To Constant : simulink/Logic and Bit Operations/Compare To Constant  relop=<=  const=0.1  OutDataTypeStr=boolean
  subjVelZeroFlg : Outport
  wires:
  Compare To Constant/1 -> subjVelZeroFlg/1
  subjVel/1 -> Abs/1
  Abs/1 -> Compare To Constant/1

== system TosAeb/FcwAebJa_Step_sys/ALG_AEBSystem/ALG_AEBInput/JaObjInfoMap/JaObjInfoMap/Subsystem/MovingStatus_Subsystem/InputMovingStatus ==
  note: 输出数值为3，类型为Moving
  note: 输出数值为2，类型为Stopped
  note: 输出数值为1，类型为Stationary
  note: 输出数值为0，类型为Unknown
  MovingStatus : Inport
  Compare To Constant : simulink/Logic and Bit Operations/Compare To Constant  relop===  const=0  OutDataTypeStr=boolean
  Compare To Constant1 : simulink/Logic and Bit Operations/Compare To Constant  relop===  const=1  OutDataTypeStr=boolean
  Compare To Constant2 : simulink/Logic and Bit Operations/Compare To Constant  relop===  const=3  OutDataTypeStr=boolean
  Compare To Constant8 : simulink/Logic and Bit Operations/Compare To Constant  relop===  const=2  OutDataTypeStr=boolean
  Input_Unknown : Outport
  Input_Stationary : Outport  Port=2
  Input_Stopped : Outport  Port=3
  Input_Moving : Outport  Port=4
  wires:
  Compare To Constant8/1 -> Input_Moving/1
  Compare To Constant1/1 -> Input_Stationary/1
  Compare To Constant2/1 -> Input_Stopped/1
  MovingStatus/1 -> Compare To Constant/1, Compare To Constant1/1, Compare To Constant2/1, Compare To Constant8/1
  Compare To Constant/1 -> Input_Unknown/1

== system TosAeb/FcwAebJa_Step_sys/ALG_AEBSystem/ALG_AEBInput/JaObjInfoMap/JaObjInfoMap/Subsystem/ObjType_Subsystem1/InputObjectType ==
  note: 输出数值为2，类型为car
  note: 输出数值为3，类型为truck
  note: 输出数值为6，类型为pedestrian行人
  note: 输出数值为4，类型为motorbike摩托车
  note: 输出数值为5，类型为bicycle自行车
  note: 输出数值为7，类型为general_object
  note: 输出数值为1，类型为unknown
  InputObjType : Inport
  Compare To Constant : simulink/Logic and Bit Operations/Compare To Constant  relop===  const=0  OutDataTypeStr=boolean
  Compare To Constant1 : simulink/Logic and Bit Operations/Compare To Constant  relop===  const=1  OutDataTypeStr=boolean
  Compare To Constant10 : simulink/Logic and Bit Operations/Compare To Constant  relop===  const=7  OutDataTypeStr=boolean
  Compare To Constant11 : simulink/Logic and Bit Operations/Compare To Constant  relop===  const=9  OutDataTypeStr=boolean
  Compare To Constant12 : simulink/Logic and Bit Operations/Compare To Constant  relop===  const=8  OutDataTypeStr=boolean
  Compare To Constant13 : simulink/Logic and Bit Operations/Compare To Constant  relop===  const=15  OutDataTypeStr=boolean
  Compare To Constant14 : simulink/Logic and Bit Operations/Compare To Constant  relop===  const=10  OutDataTypeStr=boolean
  Compare To Constant15 : simulink/Logic and Bit Operations/Compare To Constant  relop===  const=11  OutDataTypeStr=boolean
  Compare To Constant16 : simulink/Logic and Bit Operations/Compare To Constant  relop===  const=12  OutDataTypeStr=boolean
  Compare To Constant17 : simulink/Logic and Bit Operations/Compare To Constant  relop===  const=14  OutDataTypeStr=boolean
  Compare To Constant18 : simulink/Logic and Bit Operations/Compare To Constant  relop===  const=12  OutDataTypeStr=boolean
  Compare To Constant2 : simulink/Logic and Bit Operations/Compare To Constant  relop===  const=16  OutDataTypeStr=boolean
  Compare To Constant3 : simulink/Logic and Bit Operations/Compare To Constant  relop===  const=2  OutDataTypeStr=boolean
  Compare To Constant4 : simulink/Logic and Bit Operations/Compare To Constant  relop===  const=3  OutDataTypeStr=boolean
  Compare To Constant5 : simulink/Logic and Bit Operations/Compare To Constant  relop===  const=4  OutDataTypeStr=boolean
  Compare To Constant6 : simulink/Logic and Bit Operations/Compare To Constant  relop===  const=5  OutDataTypeStr=boolean
  Compare To Constant7 : simulink/Logic and Bit Operations/Compare To Constant  relop===  const=18  OutDataTypeStr=boolean
  Compare To Constant8 : simulink/Logic and Bit Operations/Compare To Constant  relop===  const=17  OutDataTypeStr=boolean
  Compare To Constant9 : simulink/Logic and Bit Operations/Compare To Constant  relop===  const=6  OutDataTypeStr=boolean
  Logical Operator : Logic  Operator=OR  Inputs=4  AllPortsSameDT=off  OutDataTypeStr=boolean
  Logical Operator1 : Logic  Operator=OR  Inputs=5  AllPortsSameDT=off  OutDataTypeStr=boolean
  Logical Operator2 : Logic  Operator=OR  AllPortsSameDT=off  OutDataTypeStr=boolean
  Logical Operator3 : Logic  Operator=OR  AllPortsSameDT=off  OutDataTypeStr=boolean
  Logical Operator5 : Logic  Operator=OR  Inputs=4  AllPortsSameDT=off  OutDataTypeStr=boolean
  Input_ObjCar : Outport
  Input_ObjTruck : Outport  Port=2
  Input_ObjPed : Outport  Port=3
  Input_ObjMotor : Outport  Port=4
  Input_ObjBicycle : Outport  Port=5
  Input_ObjGeneral : Outport  Port=6
  Input_Unknown : Outport  Port=7
  wires:
  Compare To Constant12/1 -> Logical Operator3/1
  Compare To Constant6/1 -> Logical Operator1/4
  Logical Operator/1 -> Input_ObjCar/1
  Compare To Constant13/1 -> Logical Operator3/2
  InputObjType/1 -> Compare To Constant8/1, Compare To Constant18/1, Compare To Constant17/1, Compare To Constant16/1, Compare To Constant15/1, Compare To Constant14/1, Compare To Constant13/1, Compare To Constant12/1, Compare To Constant11/1, Compare To Constant10/1, Compare To Constant9/1, Compare To Constant7/1, Compare To Constant6/1, Compare To Constant5/1, Compare To Constant4/1, Compare To Constant3/1, Compare To Constant2/1, Compare To Constant1/1, Compare To Constant/1
  Compare To Constant2/1 -> Logical Operator/3
  Compare To Constant1/1 -> Logical Operator/2
  Compare To Constant16/1 -> Logical Operator5/3
  Compare To Constant18/1 -> Input_Unknown/1
  Compare To Constant15/1 -> Logical Operator5/2
  Compare To Constant10/1 -> Logical Operator2/1
  Logical Operator5/1 -> Input_ObjGeneral/1
  Compare To Constant5/1 -> Logical Operator1/3
  Logical Operator3/1 -> Input_ObjBicycle/1
  Logical Operator1/1 -> Input_ObjTruck/1
  Compare To Constant14/1 -> Logical Operator5/1
  Compare To Constant3/1 -> Logical Operator1/1
  Logical Operator2/1 -> Input_ObjMotor/1
  Compare To Constant7/1 -> Logical Operator1/5
  Compare To Constant4/1 -> Logical Operator1/2
  Compare To Constant11/1 -> Logical Operator2/2
  Compare To Constant8/1 -> Logical Operator/4
  Compare To Constant/1 -> Logical Operator/1
  Compare To Constant17/1 -> Logical Operator5/4
  Compare To Constant9/1 -> Input_ObjPed/1

== system TosAeb/FcwAebJa_Step_sys/ALG_AEBSystem/ALG_AEBInput/JaObjInfoMap/JaObjInfoMap/Subsystem1/MovingStatus_Subsystem/InputMovingStatus ==
  note: 输出数值为3，类型为Moving
  note: 输出数值为2，类型为Stopped
  note: 输出数值为1，类型为Stationary
  note: 输出数值为0，类型为Unknown
  MovingStatus : Inport
  Compare To Constant : simulink/Logic and Bit Operations/Compare To Constant  relop===  const=0  OutDataTypeStr=boolean
  Compare To Constant1 : simulink/Logic and Bit Operations/Compare To Constant  relop===  const=1  OutDataTypeStr=boolean
  Compare To Constant2 : simulink/Logic and Bit Operations/Compare To Constant  relop===  const=3  OutDataTypeStr=boolean
  Compare To Constant8 : simulink/Logic and Bit Operations/Compare To Constant  relop===  const=2  OutDataTypeStr=boolean
  Input_Unknown : Outport
  Input_Stationary : Outport  Port=2
  Input_Stopped : Outport  Port=3
  Input_Moving : Outport  Port=4
  wires:
  Compare To Constant8/1 -> Input_Moving/1
  Compare To Constant1/1 -> Input_Stationary/1
  Compare To Constant2/1 -> Input_Stopped/1
  MovingStatus/1 -> Compare To Constant/1, Compare To Constant1/1, Compare To Constant2/1, Compare To Constant8/1
  Compare To Constant/1 -> Input_Unknown/1
